simulink model "poco_wide_11_r315"  (Simulink 7.6) (continued)
== system poco_wide_11_r315 == (continued)
      mask help: A real-sampled wideband FFT.
      mask init: same as poco_wide_11_r315/fft_wideband_real0
      settings: AttributesFormatString=|
      | 11 stages
      | (18,18)
      | Round  (unbiased: Even Values)
      | Wrap
      mask Help: eval('xlWeb(''http://casper.berkeley.edu/wiki/Fft_wideband_real'')')
      mask StyleString: edit,edit,edit,edit,checkbox,edit,edit,edit,edit,edit,edit,popup(Truncate|Round  (unbiased: +/- Inf)|Round  (unbiased: Even Values)),popup(Wrap|Saturate|Error),popup(Virtex2Pro|Virtex5),popup(logic|multipliers),edit,edit,edit,checkbox,edit,checkbox
      mask CallbackString:
      | |||||||||||||fft_callback_arch(gcb);|||||hardcode_shifts = get_param(gcb, 'hardcode_shifts');
      | set_param_state(gcb, 'shift_schedule', hardcode_shifts);||fft_callback_dsp48_adders(gcb);
      mask TabNameString: Basic,Basic,Basic,Basic,Basic,Latency,Latency,Latency,Latency,Latency,Latency,Implementation,Implementation,Implementation,Implementation,Implementation,Implementation,Implementation,Implementation,Implementation,Implementation
  fft_wideband_real3 : Subsystem  AncestorBlock=casper_library_ffts/fft_wideband_real  mask(fft_wideband_real){FFTSize=11, n_inputs=2, input_bit_width=18, coeff_bit_width=18, unscramble=on, add_latency=2, mult_latency=2, bram_latency=2, conv_latency=1, input_latency=1, biplex_direct_latency=1, quantization=Round  (unbiased: Even Values), overflow=Wrap, arch=Virtex5, opt_target=logic, coeffs_bit_limit=9, delays_bit_limit=9, mult_spec=2, hardcode_shifts=off, shift_schedule=, dsp48_adders=off}
      mask prompts: FFTSize "Size of FFT: (2^? pts)"; n_inputs "Number of Simultaneous Inputs: (2^?)"; input_bit_width "Input/Output Bit Width"; coeff_bit_width "Coefficient Bit Width"; unscramble "Unscramble output (ie, put channels in canonical order)"; add_latency "Add Latency"; mult_latency "Mult Latency"; bram_latency "BRAM Latency"; conv_latency "Convert Latency"; input_latency "Input Latency"; biplex_direct_latency "Latency between biplexes and fft_direct"; quantization "Quantization Behavior"; overflow "Overflow Behavior"; arch "Architecture"; opt_target "Use less"; coeffs_bit_limit "Number bits above which to store stage's coefficients in BRAM (2^? bits)"; delays_bit_limit "Number bits above which to store stage's delays in BRAM (2^? bits)"; mult_spec "Multiplier impl. (0=core, 1=embedded, 2=behavioural) (left=1st stage, or just one value)"; hardcode_shifts "Hardcode shift schedule"; shift_schedule "Shift schedule"; dsp48_adders "DSP48 adders in butterfly"
      mask help: A real-sampled wideband FFT.
      mask init: same as poco_wide_11_r315/fft_wideband_real0
      settings: AttributesFormatString=|
      | 11 stages
      | (18,18)
      | Round  (unbiased: Even Values)
      | Wrap
      mask Help: eval('xlWeb(''http://casper.berkeley.edu/wiki/Fft_wideband_real'')')
      mask StyleString: edit,edit,edit,edit,checkbox,edit,edit,edit,edit,edit,edit,popup(Truncate|Round  (unbiased: +/- Inf)|Round  (unbiased: Even Values)),popup(Wrap|Saturate|Error),popup(Virtex2Pro|Virtex5),popup(logic|multipliers),edit,edit,edit,checkbox,edit,checkbox
      mask CallbackString:
      | |||||||||||||fft_callback_arch(gcb);|||||hardcode_shifts = get_param(gcb, 'hardcode_shifts');
      | set_param_state(gcb, 'shift_schedule', hardcode_shifts);||fft_callback_dsp48_adders(gcb);
      mask TabNameString: Basic,Basic,Basic,Basic,Basic,Latency,Latency,Latency,Latency,Latency,Latency,Implementation,Implementation,Implementation,Implementation,Implementation,Implementation,Implementation,Implementation,Implementation,Implementation
  led0_adc_clip : xps_library/gpio  Tag=xps:gpio  SystemSampleTime=-1  io_group=ROACH:led  io_dir=out  arith_type=Boolean  bitwidth=1  bin_pt=0  bit_index=0  sample_period=1  use_single_ended=off  use_ddr=off  reg_iob=on  reg_clk_phase=0  termination=None
      link override convert: arith_type=Boolean  n_bits=1  bin_pt=0
  led1_mrst : xps_library/gpio  Tag=xps:gpio  SystemSampleTime=-1  io_group=ROACH:led  io_dir=out  arith_type=Boolean  bitwidth=1  bin_pt=0  bit_index=1  sample_period=1  use_single_ended=off  use_ddr=off  reg_iob=on  reg_clk_phase=0  termination=None
      link override convert: arith_type=Boolean  n_bits=1  bin_pt=0
  led2_sync : xps_library/gpio  Tag=xps:gpio  SystemSampleTime=-1  io_group=ROACH:led  io_dir=out  arith_type=Boolean  bitwidth=1  bin_pt=0  bit_index=2  sample_period=1  use_single_ended=off  use_ddr=off  reg_iob=on  reg_clk_phase=0  termination=None
      link override convert: arith_type=Boolean  n_bits=1  bin_pt=0
  led3_new_acc : xps_library/gpio  Tag=xps:gpio  SystemSampleTime=-1  io_group=ROACH:led  io_dir=out  arith_type=Unsigned  bitwidth=1  bin_pt=0  bit_index=3  sample_period=1  use_single_ended=off  use_ddr=off  reg_iob=on  reg_clk_phase=0  termination=None
      link override convert: arith_type=Unsigned  n_bits=1  bin_pt=0
  negedge_delay : Subsystem  AncestorBlock=casper_library_misc/negedge_delay  mask(negedge_delay){pulse_len=2^24}
      mask prompts: pulse_len "Length of Pulse"
      mask help:
      | Extends a boolean signal to be high for the specified number of clocks after
      | the last high input (negative edge).
      |
      | Minimum length is 3.
      mask init:
      | bits = ceil(log2(pulse_len+1));
      | 
      | if pulse_len < 3,
      |     errordlg('Minimum length is 3.');
      | end
  negedge_delay1 : Subsystem  AncestorBlock=casper_library_misc/negedge_delay  mask(negedge_delay){pulse_len=2^24}
      mask prompts: pulse_len "Length of Pulse"
      mask help:
      | Extends a boolean signal to be high for the specified number of clocks after
      | the last high input (negative edge).
      |
      | Minimum length is 3.
      mask init:
      | bits = ceil(log2(pulse_len+1));
      | 
      | if pulse_len < 3,
      |     errordlg('Minimum length is 3.');
      | end
  pfb_fir_real0 : Subsystem  AncestorBlock=casper_library_pfbs/pfb_fir_real  mask(pfb_fir_real){PFBSize=11, TotalTaps=4, WindowType=hamming, n_inputs=2, MakeBiplex=off, BitWidthIn=8, BitWidthOut=18, CoeffBitWidth=18, CoeffDistMem=off, add_latency=2, mult_latency=3, bram_latency=2, conv_latency=1, quantization=Round  (unbiased: Even Values), fwidth=1, mult_spec=1, adder_folding=on, adder_imp=Behavioral, coeffs_share=off}
      mask prompts: PFBSize "Size of PFB: (2^? pnts)"; TotalTaps "Total Number of Taps:"; WindowType "Windowing Function:"; n_inputs "Number of Simultaneous Inputs: (2^?)"; MakeBiplex "Make Biplex?"; BitWidthIn "Input Bitwidth:"; BitWidthOut "Output Bitwidth:"; CoeffBitWidth "Coefficient Bitwidth:"; CoeffDistMem "Use Distributed Memory for Coeffs"; add_latency "Add Latency"; mult_latency "Mult Latency"; bram_latency "BRAM Latency"; conv_latency "Convert latency"; quantization "Quantization Behavior"; fwidth "Bin Width Scaling (normal=1)"; mult_spec "Multiplier specification (0=core, 1=embedded, 2=behavioural) (left=1st tap, or just one value)"; adder_folding "Fold adders into DSPs"; adder_imp "Adder implementation"; coeffs_share "Share coefficients between polarisations (use less BRAM)?"
      mask help:
      | Fold adders into DSPs: Causes adders to be absorbed into DSP blocks (supported in Virtex5)
      | Adder implementation: Cores using Fabric or DSP48 or behavioral HDL
      mask init:
      | pfb_fir_real_init(gcb, ...
      |     'PFBSize', PFBSize, ...
      |     'TotalTaps', TotalTaps, ...
      |     'WindowType', WindowType, ...
      |     'n_inputs', n_inputs, ...
      |     'MakeBiplex', MakeBiplex, ...
      |     'BitWidthIn', BitWidthIn, ...
      |     'BitWidthOut', BitWidthOut, ...
      |     'CoeffBitWidth', CoeffBitWidth, ...
      |     'CoeffDistMem', CoeffDistMem, ...
      |     'add_latency', add_latency, ...
      |     'mult_latency', mult_latency, ...
      |     'bram_latency', bram_latency, ...
      |     'conv_latency', conv_latency, ...
      |     'quantization', quantization, ...
      |     'fwidth', fwidth, ...
      |     'mult_spec', mult_spec, ...
      |     'adder_folding', adder_folding, ...
      |     'adder_imp', adder_imp, ...
      |     'coeffs_share', coeffs_share);
      settings: AttributesFormatString=taps=4, add_latency=2
      mask Help: eval('xlWeb(''http://casper.berkeley.edu/wiki/Pfb_fir_real'')')
      mask StyleString: edit,edit,popup(bartlett|barthannwin|blackman|blackmanharris|bohamwin|chebwin|flattopwin|gausswin|hamming|hann|kaiser|nuttallwin|parzenwin|rectwin|tukeywin|triang|userwindow),edit,checkbox,edit,edit,edit,checkbox,edit,edit,edit,edit,popup(Truncate|Round  (unbiased: +/- Inf)|Round  (unbiased: Even Values)),edit,edit,checkbox,popup(Behavioral|Fabric|DSP48),checkbox
      mask CallbackString:
      | |%num_taps = eval(get_param(gcb, 'TotalTaps'));
      | %old_vec = eval(get_param(gcb, 'mult_spec'));=-0l
      | 
      | %vec = 2.*ones(1,num_taps);
      | %old_size = length(old_vec);
      | 
      | %if(old_size <= num_taps),
      | %    new_size = old_size;
      | %else,
      | %    new_size = num_taps;
      | %end
      | 
      | %for n = 1:new_size, vec(n) = old_vec(n); end
      | %set_param(gcb, 'mult_spec', mat2str(vec));|||||||||||||||||
  pfb_fir_real1 : Subsystem  AncestorBlock=casper_library_pfbs/pfb_fir_real  mask(pfb_fir_real){PFBSize=11, TotalTaps=4, WindowType=hamming, n_inputs=2, MakeBiplex=off, BitWidthIn=8, BitWidthOut=18, CoeffBitWidth=18, CoeffDistMem=off, add_latency=2, mult_latency=3, bram_latency=2, conv_latency=1, quantization=Round  (unbiased: Even Values), fwidth=1, mult_spec=1, adder_folding=on, adder_imp=DSP48, coeffs_share=off}
      mask prompts: PFBSize "Size of PFB: (2^? pnts)"; TotalTaps "Total Number of Taps:"; WindowType "Windowing Function:"; n_inputs "Number of Simultaneous Inputs: (2^?)"; MakeBiplex "Make Biplex?"; BitWidthIn "Input Bitwidth:"; BitWidthOut "Output Bitwidth:"; CoeffBitWidth "Coefficient Bitwidth:"; CoeffDistMem "Use Distributed Memory for Coeffs"; add_latency "Add Latency"; mult_latency "Mult Latency"; bram_latency "BRAM Latency"; conv_latency "Convert latency"; quantization "Quantization Behavior"; fwidth "Bin Width Scaling (normal=1)"; mult_spec "Multiplier specification (0=core, 1=embedded, 2=behavioural) (left=1st tap, or just one value)"; adder_folding "Fold adders into DSPs"; adder_imp "Adder implementation"; coeffs_share "Share coefficients between polarisations (use less BRAM)?"
      mask help:
      | Fold adders into DSPs: Causes adders to be absorbed into DSP blocks (supported in Virtex5)
      | Adder implementation: Cores using Fabric or DSP48 or behavioral HDL
      mask init: same as poco_wide_11_r315/pfb_fir_real0
      settings: AttributesFormatString=taps=4, add_latency=2
      mask Help: eval('xlWeb(''http://casper.berkeley.edu/wiki/Pfb_fir_real'')')
      mask StyleString: edit,edit,popup(bartlett|barthannwin|blackman|blackmanharris|bohamwin|chebwin|flattopwin|gausswin|hamming|hann|kaiser|nuttallwin|parzenwin|rectwin|tukeywin|triang|userwindow),edit,checkbox,edit,edit,edit,checkbox,edit,edit,edit,edit,popup(Truncate|Round  (unbiased: +/- Inf)|Round  (unbiased: Even Values)),edit,edit,checkbox,popup(Behavioral|Fabric|DSP48),checkbox
      mask CallbackString:
      | |%num_taps = eval(get_param(gcb, 'TotalTaps'));
      | %old_vec = eval(get_param(gcb, 'mult_spec'));=-0l
      | 
      | %vec = 2.*ones(1,num_taps);
      | %old_size = length(old_vec);
      | 
      | %if(old_size <= num_taps),
      | %    new_size = old_size;
      | %else,
      | %    new_size = num_taps;
      | %end
      | 
      | %for n = 1:new_size, vec(n) = old_vec(n); end
      | %set_param(gcb, 'mult_spec', mat2str(vec));|||||||||||||||||
  pfb_fir_real2 : Subsystem  AncestorBlock=casper_library_pfbs/pfb_fir_real  mask(pfb_fir_real){PFBSize=11, TotalTaps=4, WindowType=hamming, n_inputs=2, MakeBiplex=off, BitWidthIn=8, BitWidthOut=18, CoeffBitWidth=18, CoeffDistMem=off, add_latency=2, mult_latency=3, bram_latency=2, conv_latency=1, quantization=Round  (unbiased: Even Values), fwidth=1, mult_spec=1, adder_folding=on, adder_imp=DSP48, coeffs_share=off}
      mask prompts: PFBSize "Size of PFB: (2^? pnts)"; TotalTaps "Total Number of Taps:"; WindowType "Windowing Function:"; n_inputs "Number of Simultaneous Inputs: (2^?)"; MakeBiplex "Make Biplex?"; BitWidthIn "Input Bitwidth:"; BitWidthOut "Output Bitwidth:"; CoeffBitWidth "Coefficient Bitwidth:"; CoeffDistMem "Use Distributed Memory for Coeffs"; add_latency "Add Latency"; mult_latency "Mult Latency"; bram_latency "BRAM Latency"; conv_latency "Convert latency"; quantization "Quantization Behavior"; fwidth "Bin Width Scaling (normal=1)"; mult_spec "Multiplier specification (0=core, 1=embedded, 2=behavioural) (left=1st tap, or just one value)"; adder_folding "Fold adders into DSPs"; adder_imp "Adder implementation"; coeffs_share "Share coefficients between polarisations (use less BRAM)?"
      mask help:
      | Fold adders into DSPs: Causes adders to be absorbed into DSP blocks (supported in Virtex5)
      | Adder implementation: Cores using Fabric or DSP48 or behavioral HDL
      mask init: same as poco_wide_11_r315/pfb_fir_real0
      settings: AttributesFormatString=taps=4, add_latency=2
      mask Help: eval('xlWeb(''http://casper.berkeley.edu/wiki/Pfb_fir_real'')')
      mask StyleString: edit,edit,popup(bartlett|barthannwin|blackman|blackmanharris|bohamwin|chebwin|flattopwin|gausswin|hamming|hann|kaiser|nuttallwin|parzenwin|rectwin|tukeywin|triang|userwindow),edit,checkbox,edit,edit,edit,checkbox,edit,edit,edit,edit,popup(Truncate|Round  (unbiased: +/- Inf)|Round  (unbiased: Even Values)),edit,edit,checkbox,popup(Behavioral|Fabric|DSP48),checkbox
      mask CallbackString:
      | |%num_taps = eval(get_param(gcb, 'TotalTaps'));
      | %old_vec = eval(get_param(gcb, 'mult_spec'));=-0l
      | 
      | %vec = 2.*ones(1,num_taps);
      | %old_size = length(old_vec);
      | 
      | %if(old_size <= num_taps),
      | %    new_size = old_size;
      | %else,
      | %    new_size = num_taps;
      | %end
      | 
      | %for n = 1:new_size, vec(n) = old_vec(n); end
      | %set_param(gcb, 'mult_spec', mat2str(vec));|||||||||||||||||
  pfb_fir_real3 : Subsystem  AncestorBlock=casper_library_pfbs/pfb_fir_real  mask(pfb_fir_real){PFBSize=11, TotalTaps=4, WindowType=hamming, n_inputs=2, MakeBiplex=off, BitWidthIn=8, BitWidthOut=18, CoeffBitWidth=18, CoeffDistMem=off, add_latency=2, mult_latency=3, bram_latency=2, conv_latency=1, quantization=Round  (unbiased: Even Values), fwidth=1, mult_spec=1, adder_folding=on, adder_imp=DSP48, coeffs_share=off}
      mask prompts: PFBSize "Size of PFB: (2^? pnts)"; TotalTaps "Total Number of Taps:"; WindowType "Windowing Function:"; n_inputs "Number of Simultaneous Inputs: (2^?)"; MakeBiplex "Make Biplex?"; BitWidthIn "Input Bitwidth:"; BitWidthOut "Output Bitwidth:"; CoeffBitWidth "Coefficient Bitwidth:"; CoeffDistMem "Use Distributed Memory for Coeffs"; add_latency "Add Latency"; mult_latency "Mult Latency"; bram_latency "BRAM Latency"; conv_latency "Convert latency"; quantization "Quantization Behavior"; fwidth "Bin Width Scaling (normal=1)"; mult_spec "Multiplier specification (0=core, 1=embedded, 2=behavioural) (left=1st tap, or just one value)"; adder_folding "Fold adders into DSPs"; adder_imp "Adder implementation"; coeffs_share "Share coefficients between polarisations (use less BRAM)?"
      mask help:
      | Fold adders into DSPs: Causes adders to be absorbed into DSP blocks (supported in Virtex5)
      | Adder implementation: Cores using Fabric or DSP48 or behavioral HDL
      mask init: same as poco_wide_11_r315/pfb_fir_real0
      settings: AttributesFormatString=taps=4, add_latency=2
      mask Help: eval('xlWeb(''http://casper.berkeley.edu/wiki/Pfb_fir_real'')')
      mask StyleString: edit,edit,popup(bartlett|barthannwin|blackman|blackmanharris|bohamwin|chebwin|flattopwin|gausswin|hamming|hann|kaiser|nuttallwin|parzenwin|rectwin|tukeywin|triang|userwindow),edit,checkbox,edit,edit,edit,checkbox,edit,edit,edit,edit,popup(Truncate|Round  (unbiased: +/- Inf)|Round  (unbiased: Even Values)),edit,edit,checkbox,popup(Behavioral|Fabric|DSP48),checkbox
      mask CallbackString:
      | |%num_taps = eval(get_param(gcb, 'TotalTaps'));
      | %old_vec = eval(get_param(gcb, 'mult_spec'));=-0l
      | 
      | %vec = 2.*ones(1,num_taps);
      | %old_size = length(old_vec);
      | 
      | %if(old_size <= num_taps),
      | %    new_size = old_size;
      | %else,
      | %    new_size = num_taps;
      | %end
      | 
      | %for n = 1:new_size, vec(n) = old_vec(n); end
      | %set_param(gcb, 'mult_spec', mat2str(vec));|||||||||||||||||
  pol_pack0 : Subsystem
  pol_pack1 : Subsystem
  pol_pack2 : Subsystem
  pol_pack3 : Subsystem
  pulse_ext2 : Subsystem  mask{pulse_len=2^24}
      mask prompts: pulse_len "Length of Pulse"
      mask init:
      | bits = ceil(log2(pulse_len+1));
  pulse_ext6 : Subsystem  mask{pulse_len=2^24}
      mask prompts: pulse_len "Length of Pulse"
      mask init:
      | bits = ceil(log2(pulse_len+1));
  quant0 : Subsystem
  quant1 : Subsystem
  quant2 : Subsystem
  quant3 : Subsystem
  snap_adc0 : Subsystem  AncestorBlock=casper_library/Scopes/snap  mask(snap){nsamples=11}
      mask prompts: nsamples "No. of samples(2^?)"
      mask help:
      | The <i>snap</i> block provides a packaged solution to capturing data from
      | the FPGA fabric and making it accessible from the CPU.  The block comes in
      | 2 flavors: <i>snap</i> (which captures to a 32 bit wide Shared BRAM), and
      | <i>snap64</i> (which captures to 2x32 bit wide Shared BRAMs to effect a
      | 64 bit capture).
      mask Help: eval('xlWeb([getenv(''MLIB_ROOT''), ''\doc\htmlblocks\sys_snap\sys_snap.html''])')
  snap_adc1 : Subsystem  AncestorBlock=casper_library/Scopes/snap  mask(snap){nsamples=11}
      mask prompts: nsamples "No. of samples(2^?)"
      mask help:
      | The <i>snap</i> block provides a packaged solution to capturing data from
      | the FPGA fabric and making it accessible from the CPU.  The block comes in
      | 2 flavors: <i>snap</i> (which captures to a 32 bit wide Shared BRAM), and
      | <i>snap64</i> (which captures to 2x32 bit wide Shared BRAMs to effect a
      | 64 bit capture).
      mask Help: eval('xlWeb([getenv(''MLIB_ROOT''), ''\doc\htmlblocks\sys_snap\sys_snap.html''])')
  snap_adc2 : Subsystem  AncestorBlock=casper_library/Scopes/snap  mask(snap){nsamples=11}
      mask prompts: nsamples "No. of samples(2^?)"
      mask help:
      | The <i>snap</i> block provides a packaged solution to capturing data from
      | the FPGA fabric and making it accessible from the CPU.  The block comes in
      | 2 flavors: <i>snap</i> (which captures to a 32 bit wide Shared BRAM), and
      | <i>snap64</i> (which captures to 2x32 bit wide Shared BRAMs to effect a
      | 64 bit capture).
      mask Help: eval('xlWeb([getenv(''MLIB_ROOT''), ''\doc\htmlblocks\sys_snap\sys_snap.html''])')
  snap_adc3 : Subsystem  AncestorBlock=casper_library/Scopes/snap  mask(snap){nsamples=11}
      mask prompts: nsamples "No. of samples(2^?)"
      mask help:
      | The <i>snap</i> block provides a packaged solution to capturing data from
      | the FPGA fabric and making it accessible from the CPU.  The block comes in
      | 2 flavors: <i>snap</i> (which captures to a 32 bit wide Shared BRAM), and
      | <i>snap64</i> (which captures to 2x32 bit wide Shared BRAMs to effect a
      | 64 bit capture).
      mask Help: eval('xlWeb([getenv(''MLIB_ROOT''), ''\doc\htmlblocks\sys_snap\sys_snap.html''])')
  status : xps_library/software register  Tag=xps:sw_reg  SystemSampleTime=-1  io_dir=To Processor  arith_type=Unsigned  bitwidth=32  bin_pt=0  sample_period=1  gw_name=poco_wide_11_r315_status_user_data_in
      link override convert: arith_type=Unsigned  n_bits=32  bin_pt=0
  sync_gen : Subsystem  mask(sync_gen_hw){sync_period_bits=27}
      mask prompts: sync_period_bits "Size of sync generator counter (bits)"
      mask help:
      | This circuit implements a scheme for synchronizing a design to within an FPGA clock of an external 1 pulse-per-second signal.
      |
      | * An arm pulse will cause the sync_gen to stop issuing sync pulses.
      | * It will then ignore the next input trigger (1PPS), while sync is flushed through the DSP pipeline.
      | * It will then lock onto the next trigger (1PPS) and issue syncs thereafter every sync_period clocks.
      | * You will need a multiple of (pfb_fir taps*LCM(FFT reorder orders) *fft bins/parallel inputs). See the sync memo for further details.
  wires:
  adc1/16 -> Logical/4
  adc1/15 -> Logical/3
  adc1/14 -> Logical/2
  adc1/13 -> Logical/1
  Logical/1 -> Delay9/1
  adc1/9 -> Logical2/1
  adc1/10 -> Logical2/2
  adc1/11 -> Logical1/1
  adc1/12 -> Logical1/2
  Logical2/1 -> Delay2/1
  adc1/5 -> pol_pack1/1(In1)
  adc1/6 -> pol_pack1/2(In2)
  adc1/7 -> pol_pack1/3(In3)
  adc1/8 -> pol_pack1/4(In4)
  pol_pack1/1(Out1) -> Delay1/1
  adc1/1 -> pol_pack0/1(In1)
  adc1/2 -> pol_pack0/2(In2)
  adc1/3 -> pol_pack0/3(In3)
  adc1/4 -> pol_pack0/4(In4)
  pol_pack0/1(Out1) -> Delay/1
  Pulse Generator/1 -> adc1/3
  Pulse Generator1/1 -> adc1/1
  Pulse Generator2/1 -> adc1/2
  Constant/1 -> adc1/4
  [adc0_sync] -> Logical3/2
  sync_gen/1(mrst) -> [mrst]
  sync_gen/2(sync) -> [timing_sync]
  Logical3/1 -> sync_gen/2(pps)
  [soft_sync] -> Logical3/1
  Constant9/1 -> fft_shift/1
  fft_shift/1 -> Slice6/1
  pfb_fir_real0/2(pol1_out1) -> a0p0_1st_shift/2(din1)
  pfb_fir_real0/3(pol1_out2) -> a0p0_1st_shift/3(din2)
  pfb_fir_real0/4(pol1_out3) -> a0p0_1st_shift/4(din3)
  pfb_fir_real0/5(pol1_out4) -> a0p0_1st_shift/5(din4)
  a0p0_1st_shift/2(dout1) -> fft_wideband_real0/3(in0)
  a0p0_1st_shift/3(dout2) -> fft_wideband_real0/4(in1)
  a0p0_1st_shift/4(dout3) -> fft_wideband_real0/5(in2)
  a0p0_1st_shift/5(dout4) -> fft_wideband_real0/6(in3)
  Slice6/1 -> fft_wideband_real2/2(shift), fft_wideband_real3/2(shift), fft_wideband_real1/2(shift), fft_wideband_real0/2(shift)
  pfb_fir_real0/1(sync_out) -> a0p0_1st_shift/1(sync_in)
  a0p0_1st_shift/1(sync_out) -> fft_wideband_real0/1(sync)
  fft_wideband_real0/4(of) -> [fft_over0]
  [adc0_pol0] -> adc_unpack0/1(In1)
  adc2/16 -> Logical6/4
  adc2/15 -> Logical6/3
  adc2/14 -> Logical6/2
  adc2/13 -> Logical6/1
  Logical6/1 -> Delay14/1
  Pulse Generator3/1 -> adc2/3
  Pulse Generator4/1 -> adc2/1
  Pulse Generator5/1 -> adc2/2
  Constant39/1 -> adc2/4
  pfb_fir_real1/2(pol1_out1) -> a0p1_1st_shift/2(din1)
  pfb_fir_real1/3(pol1_out2) -> a0p1_1st_shift/3(din2)
  pfb_fir_real1/4(pol1_out3) -> a0p1_1st_shift/4(din3)
  pfb_fir_real1/5(pol1_out4) -> a0p1_1st_shift/5(din4)
  a0p1_1st_shift/2(dout1) -> fft_wideband_real1/3(in0)
  a0p1_1st_shift/3(dout2) -> fft_wideband_real1/4(in1)
  a0p1_1st_shift/4(dout3) -> fft_wideband_real1/5(in2)
  a0p1_1st_shift/5(dout4) -> fft_wideband_real1/6(in3)
  pfb_fir_real1/1(sync_out) -> a0p1_1st_shift/1(sync_in)
  a0p1_1st_shift/1(sync_out) -> fft_wideband_real1/1(sync)
  fft_wideband_real1/4(of) -> [fft_over1]
  pfb_fir_real2/2(pol1_out1) -> a1p0_1st_shift/2(din1)
  pfb_fir_real2/3(pol1_out2) -> a1p0_1st_shift/3(din2)
  pfb_fir_real2/4(pol1_out3) -> a1p0_1st_shift/4(din3)
  pfb_fir_real2/5(pol1_out4) -> a1p0_1st_shift/5(din4)
  a1p0_1st_shift/2(dout1) -> fft_wideband_real2/3(in0)
  a1p0_1st_shift/3(dout2) -> fft_wideband_real2/4(in1)
  a1p0_1st_shift/4(dout3) -> fft_wideband_real2/5(in2)
  a1p0_1st_shift/5(dout4) -> fft_wideband_real2/6(in3)
  pfb_fir_real2/1(sync_out) -> a1p0_1st_shift/1(sync_in)
  a1p0_1st_shift/1(sync_out) -> fft_wideband_real2/1(sync)
  [timing_sync] -> pfb_fir_real3/1(sync)
  pfb_fir_real3/2(pol1_out1) -> a1p1_1st_shift/2(din1)
  pfb_fir_real3/3(pol1_out2) -> a1p1_1st_shift/3(din2)
  pfb_fir_real3/4(pol1_out3) -> a1p1_1st_shift/4(din3)
  pfb_fir_real3/5(pol1_out4) -> a1p1_1st_shift/5(din4)
  a1p1_1st_shift/2(dout1) -> fft_wideband_real3/3(in0)
  a1p1_1st_shift/3(dout2) -> fft_wideband_real3/4(in1)
  a1p1_1st_shift/4(dout3) -> fft_wideband_real3/5(in2)
  a1p1_1st_shift/5(dout4) -> fft_wideband_real3/6(in3)
  pfb_fir_real3/1(sync_out) -> a1p1_1st_shift/1(sync_in)
  a1p1_1st_shift/1(sync_out) -> fft_wideband_real3/1(sync)
  Logical1/1 -> Delay8/1
  adc_unpack0/1(Out1) -> pfb_fir_real0/2(pol1_in1)
  adc_unpack0/2(Out2) -> pfb_fir_real0/3(pol1_in2)
  adc_unpack0/3(Out3) -> pfb_fir_real0/4(pol1_in3)
  adc_unpack0/4(Out4) -> pfb_fir_real0/5(pol1_in4)
  quant0/1(sync_out) -> [sync_rnd]
  quant0/3(dout0) -> [eq0]
  quant0/4(dout1) -> [eq1]
  Constant11/1 -> acc_len/1
  acc_len/1 -> acc_ctrl/3(acc_len)
  Concat6/1 -> Delay21/1
  Slice12/1 -> dir_x0/2(a)
  Slice13/1 -> dir_x0/3(b)
  Slice15/1 -> dir_x0/4(c)
  Slice20/1 -> dir_x0/5(d)
  Counter1/1 -> acc_num/1
  acc_ctrl/1(new_acc) -> Delay6/1, Delay7/1
  [mrst] -> acc_ctrl/1(mrst)
  Delay5/1 -> [new_acc]
  [sync_rnd] -> acc_ctrl/2(sync)
  acc_num/1 -> Scope2/1
  Delay3/1 -> Counter1/2
  [mrst] -> Counter1/1
  Delay6/1 -> dir_x0/1(new_acc), Delay3/1, Delay5/1
  [eq0] -> Concat6/1
  [eq2] -> Concat6/2
  [eq4] -> Concat6/3
  [eq6] -> Concat6/4
  [eq1] -> Concat7/1
  [eq3] -> Concat7/2
  [eq5] -> Concat7/3
  [eq7] -> Concat7/4
  fft_wideband_real2/4(of) -> [fft_over2]
  Delay20/1 -> Slice37/1, Slice38/1, Slice39/1, Slice36/1
  Slice36/1 -> dir_x1/2(a)
  Slice37/1 -> dir_x1/3(b)
  Slice38/1 -> dir_x1/4(c)
  Slice39/1 -> dir_x1/5(d)
  Concat7/1 -> Delay20/1
  Delay7/1 -> dir_x1/1(new_acc)
  [adc1_sync] -> Logical3/3
  Constant1/1 -> ctrl/1
  ctrl/1 -> Slice26/1, Slice31/1, Slice32/1
  adc1/17 -> Terminator5/1
  adc2/17 -> Terminator4/1
  [clip_rst] -> clip_detect/1(rst)
  [adc_over3] -> clip_detect/30(In_03)
  [adc_over2] -> clip_detect/31(In_02)
  [adc_over1] -> clip_detect/32(In_01)
  [adc_over0] -> clip_detect/33(in_lsb)
  Constant16/1 -> clip_detect/29(In_04)
  Constant17/1 -> clip_detect/26(In_07)
  Constant18/1 -> clip_detect/27(In_06)
  Constant13/1 -> clip_detect/28(In_05)
  [fft_over0] -> clip_detect/25(In_08)
  [fft_over1] -> clip_detect/24(In_09)
  [fft_over2] -> clip_detect/23(In_10)
  [fft_over3] -> clip_detect/22(In_11)
  [mrst] -> clip_detect/2(in_msb_nr)
  [timing_sync] -> clip_detect/3(In_30_nr)
  [new_acc] -> clip_detect/4(In_29_nr)
  Constant14/1 -> clip_detect/5(In_28_nr)
  Constant19/1 -> clip_detect/6(In_27_nr)
  Constant2/1 -> clip_detect/7(In_26_nr)
  Constant20/1 -> clip_detect/8(In_25_nr)
  Constant21/1 -> clip_detect/9(In_24_nr)
  [eq_clip3] -> clip_detect/14(In_19)
  [eq_clip2] -> clip_detect/15(In_18)
  [eq_clip1] -> clip_detect/16(In_17)
  [eq_clip0] -> clip_detect/17(In_16)
  Constant7/1 -> clip_detect/18(In_15)
  Constant8/1 -> clip_detect/19(In_14)
  Constant10/1 -> clip_detect/20(In_13)
  Constant12/1 -> clip_detect/21(In_12)
  clip_detect/1(registered_out) -> status/1
  Slice26/1 -> edge_detect/1(in)
  [adc0_pol0] -> decat0/1(In1)
  Slice31/1 -> edge_detect1/1(in)
  Slice32/1 -> edge_detect2/1(in)
  edge_detect1/1(out) -> Delay15/1
  edge_detect/1(out) -> Delay16/1
  edge_detect2/1(out) -> Delay4/1
  [sync_arm] -> sync_gen/1(arm)
  Delay/1 -> [adc0_pol0]
  Delay1/1 -> [adc0_pol1]
  Delay2/1 -> [adc_over0]
  Delay8/1 -> [adc_over1]
  Delay9/1 -> [adc0_sync]
  Delay10/1 -> [adc1_pol0]
  Delay11/1 -> [adc1_pol1]
  Delay12/1 -> [adc_over2]
  Delay13/1 -> [adc_over3]
  Delay14/1 -> [adc1_sync]
  Delay21/1 -> Slice12/1, Slice13/1, Slice15/1, Slice20/1
  pulse_ext2/1(out) -> Inverter2/1
  [timing_sync] -> pulse_ext2/1(in)
  [mrst] -> negedge_delay1/1(in)
  pulse_ext6/1(out) -> Inverter3/1
  [new_acc] -> pulse_ext6/1(in)
  led0_adc_clip/1 -> Terminator1/1
  led1_mrst/1 -> Terminator2/1
  led2_sync/1 -> Terminator12/1
  led3_new_acc/1 -> Terminator13/1
  Logical4/1 -> negedge_delay/1(in)
  negedge_delay/1(out) -> Inverter/1
  [adc_over0] -> Logical4/1
  [adc_over1] -> Logical4/2
  [adc_over2] -> Logical4/3
  [adc_over3] -> Logical4/4
  negedge_delay1/1(out) -> Inverter1/1
  status/1 -> Terminator3/1
  [timing_sync] -> pfb_fir_real0/1(sync)
  [timing_sync] -> pfb_fir_real1/1(sync)
  Constant15/1 -> clip_detect/10(In_23)
  Constant3/1 -> clip_detect/11(In_22)
  Constant4/1 -> clip_detect/12(In_21)
  Constant5/1 -> clip_detect/13(In_20)
  quant0/2(clip_out) -> [eq_clip0]
  fft_wideband_real0/2(out0) -> quant0/2(din0)
  fft_wideband_real0/3(out1) -> quant0/3(din1)
  fft_wideband_real0/1(sync_out) -> quant0/1(sync)
  quant1/1(sync_out) -> Terminator8/1
  quant1/3(dout0) -> [eq2]
  quant1/4(dout1) -> [eq3]
  quant1/2(clip_out) -> [eq_clip1]
  quant2/1(sync_out) -> Terminator7/1
  quant2/3(dout0) -> [eq4]
  quant2/2(clip_out) -> [eq_clip2]
  quant3/1(sync_out) -> Terminator6/1
  quant3/3(dout0) -> [eq6]
  quant3/4(dout1) -> [eq7]
  quant3/2(clip_out) -> [eq_clip3]
  Delay4/1 -> [soft_sync]
  Delay15/1 -> [sync_arm]
  Delay16/1 -> [clip_rst]
  Inverter/1 -> led0_adc_clip/1
  Inverter1/1 -> led1_mrst/1
  Inverter2/1 -> led2_sync/1
  Inverter3/1 -> led3_new_acc/1
  [timing_sync] -> pfb_fir_real2/1(sync)
  adc2/9 -> Logical9/1
  adc2/10 -> Logical9/2
  adc2/11 -> Logical8/1
  adc2/12 -> Logical8/2
  Logical9/1 -> Delay12/1
  Logical8/1 -> Delay13/1
  adc2/5 -> pol_pack3/1(In1)
  adc2/6 -> pol_pack3/2(In2)
  adc2/7 -> pol_pack3/3(In3)
  adc2/8 -> pol_pack3/4(In4)
  pol_pack3/1(Out1) -> Delay11/1
  adc2/1 -> pol_pack2/1(In1)
  adc2/2 -> pol_pack2/2(In2)
  adc2/3 -> pol_pack2/3(In3)
  adc2/4 -> pol_pack2/4(In4)
  pol_pack2/1(Out1) -> Delay10/1
  calc_adc_sum_sq/1(sum_sq) -> adc_sum_sq0/1
  decat0/1(Out1) -> calc_adc_sum_sq/1(In1)
  decat0/2(Out2) -> calc_adc_sum_sq/2(In2)
  decat0/3(Out3) -> calc_adc_sum_sq/3(In3)
  decat0/4(Out4) -> calc_adc_sum_sq/4(In4)
  adc_sum_sq0/1 -> Terminator/1
  calc_adc_sum_sq4/1(sum_sq) -> adc_sum_sq1/1
  decat1/1(Out1) -> calc_adc_sum_sq4/1(In1)
  decat1/2(Out2) -> calc_adc_sum_sq4/2(In2)
  decat1/3(Out3) -> calc_adc_sum_sq4/3(In3)
  decat1/4(Out4) -> calc_adc_sum_sq4/4(In4)
  adc_sum_sq1/1 -> Terminator25/1
  calc_adc_sum_sq5/1(sum_sq) -> adc_sum_sq2/1
  decat2/1(Out1) -> calc_adc_sum_sq5/1(In1)
  decat2/2(Out2) -> calc_adc_sum_sq5/2(In2)
  decat2/3(Out3) -> calc_adc_sum_sq5/3(In3)
  decat2/4(Out4) -> calc_adc_sum_sq5/4(In4)
  adc_sum_sq2/1 -> Terminator30/1
  calc_adc_sum_sq6/1(sum_sq) -> adc_sum_sq3/1
  decat3/1(Out1) -> calc_adc_sum_sq6/1(In1)
  decat3/2(Out2) -> calc_adc_sum_sq6/2(In2)
  decat3/3(Out3) -> calc_adc_sum_sq6/3(In3)
  decat3/4(Out4) -> calc_adc_sum_sq6/4(In4)
  adc_sum_sq3/1 -> Terminator31/1
  [adc0_pol1] -> decat1/1(In1)
  [adc1_pol0] -> decat2/1(In1)
  [adc1_pol1] -> decat3/1(In1)
  adc_unpack1/1(Out1) -> pfb_fir_real1/2(pol1_in1)
  adc_unpack1/2(Out2) -> pfb_fir_real1/3(pol1_in2)
  adc_unpack1/3(Out3) -> pfb_fir_real1/4(pol1_in3)
  adc_unpack1/4(Out4) -> pfb_fir_real1/5(pol1_in4)
  [adc0_pol1] -> adc_unpack1/1(In1)
  adc_unpack2/1(Out1) -> pfb_fir_real2/2(pol1_in1)
  adc_unpack2/2(Out2) -> pfb_fir_real2/3(pol1_in2)
  adc_unpack2/3(Out3) -> pfb_fir_real2/4(pol1_in3)
  adc_unpack2/4(Out4) -> pfb_fir_real2/5(pol1_in4)
  [adc1_pol0] -> adc_unpack2/1(In1)
  [adc1_pol1] -> adc_unpack3/1(In1)
  adc_unpack3/1(Out1) -> pfb_fir_real3/2(pol1_in1)
  adc_unpack3/2(Out2) -> pfb_fir_real3/3(pol1_in2)
  adc_unpack3/3(Out3) -> pfb_fir_real3/4(pol1_in3)
  adc_unpack3/4(Out4) -> pfb_fir_real3/5(pol1_in4)
  fft_wideband_real1/2(out0) -> quant1/2(din0)
  fft_wideband_real1/3(out1) -> quant1/3(din1)
  fft_wideband_real2/1(sync_out) -> quant2/1(sync)
  fft_wideband_real2/2(out0) -> quant2/2(din0)
  fft_wideband_real2/3(out1) -> quant2/3(din1)
  fft_wideband_real3/1(sync_out) -> quant3/1(sync)
  fft_wideband_real3/2(out0) -> quant3/2(din0)
  fft_wideband_real3/3(out1) -> quant3/3(din1)
  quant2/4(dout1) -> [eq5]
  [adc0_pol0] -> snap_adc0/1(din)
  Constant6/1 -> snap_adc0/2(trig), snap_adc0/3(we)
  [adc0_pol1] -> snap_adc1/1(din)
  Constant25/1 -> snap_adc1/3(we), snap_adc1/2(trig)
  [adc1_pol0] -> snap_adc2/1(din)
  Constant26/1 -> snap_adc2/2(trig), snap_adc2/3(we)
  [adc1_pol1] -> snap_adc3/1(din)
  Constant27/1 -> snap_adc3/3(we), snap_adc3/2(trig)
  fft_wideband_real1/1(sync_out) -> quant1/1(sync)
  fft_wideband_real3/4(of) -> [fft_over3]
